simulink model "PrkgClimaPreProcMgr"  (R2022b)
settings: Solver=FixedStepDiscrete  StartTime=0.0  StopTime=10.0  FixedStep=0.2  RelTol=1e-3
(part 1 of 2)
settings Model: Creator=blue.ge  LastModifiedBy=blue.ge
settings DataIOCC: SaveFormat=StructureWithTime
settings OptimizationCC: DefaultParameterBehavior=Inlined  EnhancedBackFolding=on  PassReuseOutputArgsAs=Individual arguments  ZeroExternalMemoryAtStartup=off  ZeroInternalMemoryAtStartup=off  OptimizeBlockOrder=speed  DifferentSizesBufferReuse=on  OptimizationCustomize=off  EfficientTunableParamExpr=on
settings DebuggingCC: ParameterTunabilityLossMsg=error
settings ModelReferenceCC: ModelReferenceNumInstancesAllowed=Single
settings SFSimCC: MATLABDynamicMemAlloc=off
settings RTWCC: Description=AUTOSAR  SystemTargetFile=autosar.tlc  GenCodeOnly=on  TemplateMakefile=ert_default_tmf
settings CodeAppCC: ForceParamTrailComments=on  IgnoreCustomStorageClasses=off  OperatorAnnotations=on  SimulinkDataObjDesc=on  SFDataObjDesc=on  InsertBlockDesc=on
settings STFCustomTargetCC: TargetLangStandard=C99 (ISO)  MultiwordLength=256  ParMdlRefBuildCompliant=on  CompOptLevelCompliant=on  IncludeMdlTerminateFcn=off  CombineOutputUpdateFcns=on  SuppressErrorStatus=on  ERTCustomFileBanners=on  SupportNonFinite=off  SupportComplex=off  SupportContinuousTime=off  SupportNonInlinedSFcns=off  RemoveResetFunc=on  MATLABClassNameForMDSCustomization=Simulink.SoftwareTarget.ERTCustomization  AutosarCompliant=on  UseToolchainInfoCompliant=on  LookupTableObjectStructAxisOrder=2,1,3,4,...  LUTObjectStructOrderEvenSpacing=Size,Table,Breakpoints  SystemTargetFile=autosar.tlc
settings ERTTargetCC: TargetLangStandard=C99 (ISO)  ConcurrentExecutionCompliant=off  IncludeMdlTerminateFcn=off  SuppressErrorStatus=on  SupportNonFinite=off  SupportComplex=off  ModelStepFunctionPrototypeControlCompliant=off  CPPClassGenCompliant=off  AutosarCompliant=on  LookupTableObjectStructAxisOrder=2,1,3,4,...  LUTObjectStructOrderEvenSpacing=Size,Table,Breakpoints  InlinedParameterPlacement=NonHierarchical  ERTCustomFileTemplate=example_file_process.tlc

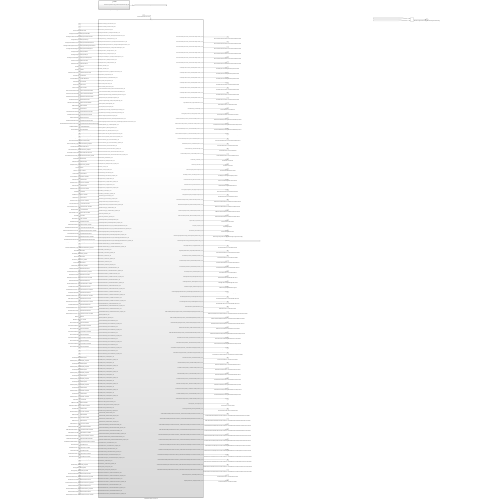
[thumbnail: Simulink's stored preview of the top-level diagram]
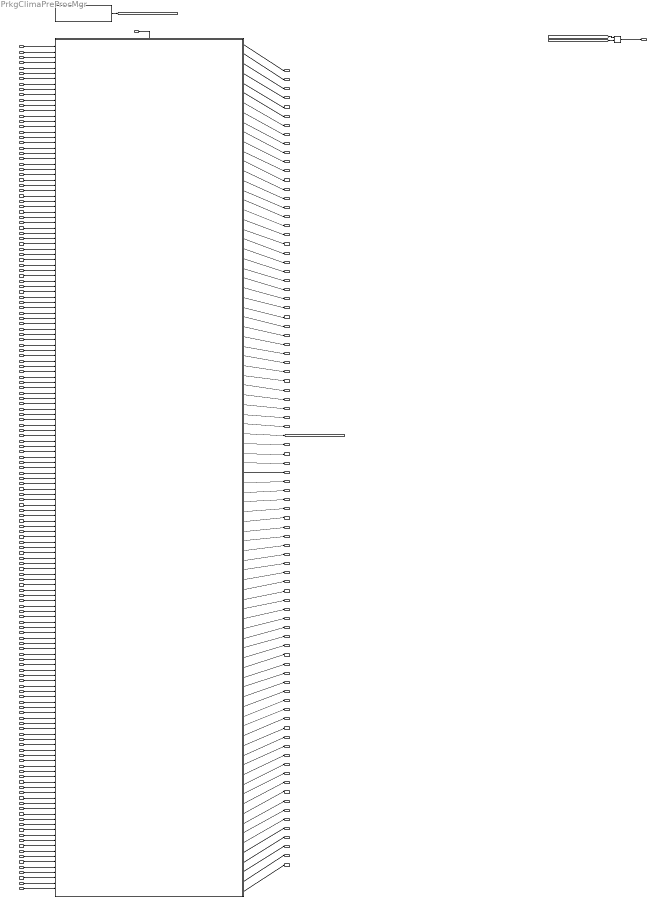
== system PrkgClimaPreProcMgr ==
  PrkgClimaPreProcMgr_Runnable : Inport  SampleTime=0.2  OutputFunctionCall=on
  AmbTFildForClimaNgcc_AmbTFildForClima : Inport  Port=2  OutDataTypeStr=T30  PortDimensions=1
  AmbTFildForClimaQf_AmbTFildForClimaQf : Inport  Port=3  OutDataTypeStr=Enum: GenQf1  PortDimensions=1
  CarTiIntForClima_CarTiIntForClima : Inport  Port=4  OutDataTypeStr=CarTiGlb  PortDimensions=1
  ClimaCfgForClimaCtrlMan1_ClimaCfgForClimaCtrlMan1 : Inport  Port=5  OutDataTypeStr=Boolean  PortDimensions=1
  ClimaCfgForComIfGenn2ForTelm1_ClimaCfgForComIfGenn2ForTelm1 : Inport  Port=6  OutDataTypeStr=Boolean  PortDimensions=1
  ClimaCfgForHeatrTyp1_ClimaCfgForHeatrTyp1 : Inport  Port=7  OutDataTypeStr=Enum: ClimaCfgForHeatrTyp1  PortDimensions=1
  ClimaCfgForHvacHeatrElecFrntPrsnt1_ClimaCfgForHvacHeatrElecFrntPrsnt1 : Inport  Port=8  OutDataTypeStr=Boolean  PortDimensions=1
  ClimaCfgForSeatHeatgForRowFirstPrsnt1_ClimaCfgForSeatHeatgForRowFirstPrsnt1 : Inport  Port=9  OutDataTypeStr=Boolean  PortDimensions=1
  ClimaCfgForSteerWhlHeatgPrsnt1_ClimaCfgForSteerWhlHeatgPrsnt1 : Inport  Port=10  OutDataTypeStr=Boolean  PortDimensions=1
  ClimaCfgForVehElec1_ClimaCfgForVehElec1 : Inport  Port=11  OutDataTypeStr=Boolean  PortDimensions=1
  ClimaCfgForVehHyb1_ClimaCfgForVehHyb1 : Inport  Port=12  OutDataTypeStr=Boolean  PortDimensions=1
  ClimaCfgForVrntOfVehSysElec1_ClimaCfgForVrntOfVehSysElec1 : Inport  Port=13  OutDataTypeStr=Enum: ClimaCenCfgForVrntOfVehSysElec1  PortDimensions=1
  ClimaRqrd1ConnGen2_ClimaRqrd1ConnGen2 : Inport  Port=14  OutDataTypeStr=Enum: OnOffNoReq  PortDimensions=1
  ClimaRqrdFromRem1_ClimaRqrdFromRem1 : Inport  Port=15  OutDataTypeStr=Enum: OnOffNoReq  PortDimensions=1
  ClimaStrtTi_ClimaStrtTi : Inport  Port=16  OutDataTypeStr=CarTiGlb  PortDimensions=1
  ClimaWarn_ClimaWarn : Inport  Port=17  OutDataTypeStr=Enum: ClimaWarn  PortDimensions=1
  ClngRqrdFromTelmConnGen2_ClngRqrdFromTelmConnGen2 : Inport  Port=18  OutDataTypeStr=Enum: OnOffNoReq  PortDimensions=1
  CmptmtObsvrSts_CmptmtObsvrSts : Inport  Port=19  OutDataTypeStr=Enum: ObsvrStsForCmptmt  PortDimensions=1
  CmptmtSunMtrlTEstimd_CmptmtSunMtrlTEstimd : Inport  Port=20  OutDataTypeStr=T30  PortDimensions=1
  DCChrgnHndlSts_DCChrgnHndlSts : Inport  Port=21  OutDataTypeStr=Enum: DCChrgnHndlStsE2E  PortDimensions=1
  DshbTForClima_DshbTForClima : Inport  Port=22  OutDataTypeStr=T30  PortDimensions=1
  DshbTForClimaQf_DshbTForClimaQf : Inport  Port=23  OutDataTypeStr=Enum: DshbTForClimaQf  PortDimensions=1
  HvacAirTForRowFirstAtVentLeRaw_HvacAirTForRowFirstAtVentLeRaw : Inport  Port=24  OutDataTypeStr=Bus: HvacAirTForRowFirstAtVentLe  PortDimensions=1  BusOutputAsStruct=on
  HvacAirTForRowFirstAtVentRiRaw_HvacAirTForRowFirstAtVentRiRaw : Inport  Port=25  OutDataTypeStr=Bus: HvacAirTForRowFirstAtVentRi  PortDimensions=1  BusOutputAsStruct=on
  MemReadForPrkgClimaPreProcMgr_MemReadForPrkgClimaPreProcMgr : Inport  Port=26  OutDataTypeStr=Bus: MemRecForPrkgClimaPreProcMgr  PortDimensions=1  BusOutputAsStruct=on
  MemReqStsForPrkgClima_MemReqStsForPrkgClima : Inport  Port=27  OutDataTypeStr=Enum: MemReqSts  PortDimensions=1
  NetReqFromPrkgClimaEveMgr_NetReqFromPrkgClimaEveMgr : Inport  Port=28  OutDataTypeStr=Boolean  PortDimensions=1
  OnBdChrgrHndlSts_OnBdChrgrHndlSts : Inport  Port=29  OutDataTypeStr=Enum: OnBdChrgrSts1  PortDimensions=1
  ReqForPrkgClima_ReqForPrkgClima : Inport  Port=30  OutDataTypeStr=Enum: PrkgClimaReqSts  PortDimensions=1
  ReqForSwtHeatrTypFromActvMgr_ReqForSwtHeatrTypFromActvMgr : Inport  Port=31  OutDataTypeStr=Boolean  PortDimensions=1
  ReqForSwtHeatrTypFromEveMgr_ReqForSwtHeatrTypFromEveMgr : Inport  Port=32  OutDataTypeStr=Boolean  PortDimensions=1
  RtMaxForPrePrkgClima_RtMaxForPrePrkgClima : Inport  Port=33  OutDataTypeStr=Ti33  PortDimensions=1
  SeatHeatDurgClimaEnadConnGen2_SeatHeatDurgClimaEnadConnGen2 : Inport  Port=34  OutDataTypeStr=Enum: SeatHeatDurgClimaEnad  PortDimensions=1
  SteerWhlHeatgDurgClimEandFromTelmConnGen2_SteerWhlHeatgDurgClimEandFromTelmConnGen2 : Inport  Port=35  OutDataTypeStr=Enum: OnOff1  PortDimensions=1
  VehModMngtGlbSafe1_Ngcc_VehModMngtGlbSafe1 : Inport  Port=36  OutDataTypeStr=Bus: VehModMngtGlbSafe1  PortDimensions=1  BusOutputAsStruct=on
  WarnForEgyAllwdLimd_WarnForEgyAllwdLimd : Inport  Port=37  OutDataTypeStr=Boolean  PortDimensions=1
  WarnForHybWoUForTmr_WarnForHybWoUForTmr : Inport  Port=38  OutDataTypeStr=Boolean  PortDimensions=1
  WarnForHybWoUFromActvMgr_WarnForHybWoUFromActvMgr : Inport  Port=39  OutDataTypeStr=Boolean  PortDimensions=1
  WarnForTmrPreCdngFctNotAvl_WarnForTmrPreCdngFctNotAvl : Inport  Port=40  OutDataTypeStr=Boolean  PortDimensions=1
  BookTrvlClimaActvReq_Ngcc_BookTrvlClimaActvReq : Inport  Port=41  OutDataTypeStr=Enum: OnOffNoReq  PortDimensions=1
  BookTrvlClimaActvReq_Ngcc_BookTrvlClimaActvReq_IsUpdated : Inport  Port=42  OutDataTypeStr=boolean  PortDimensions=1
  CbnOverHeatProtnEna_CbnOverHeatProtnEna : Inport  Port=43  OutDataTypeStr=Enum: OnOff1  PortDimensions=1
  CbnOverHeatProtnEna_CbnOverHeatProtnEna_IsUpdated : Inport  Port=44  OutDataTypeStr=boolean  PortDimensions=1
  CbnOverHeatProtnEnaFromTelm_CbnOverHeatProtnEnaFromTelm : Inport  Port=45  OutDataTypeStr=Enum: OnOff1  PortDimensions=1
  CbnOverHeatProtnEnaFromTelm_CbnOverHeatProtnEnaFromTelm_IsUpdated : Inport  Port=46  OutDataTypeStr=boolean  PortDimensions=1
  CdsClimaOnReq_CdsClimaOnReq : Inport  Port=47  OutDataTypeStr=Enum: OnOff1  PortDimensions=1
  ClimaRqrdFromHmi_ClimaRqrdFromHmi : Inport  Port=48  OutDataTypeStr=Enum: OnOffNoReq  PortDimensions=1
  ClimaRqrdFromHmi_ClimaRqrdFromHmi_IsUpdated : Inport  Port=49  OutDataTypeStr=boolean  PortDimensions=1
  ClimaTmr_ClimaTmr : Inport  Port=50  OutDataTypeStr=CarTiGlb  PortDimensions=1
  ClimaTmr_ClimaTmr_IsUpdated : Inport  Port=51  OutDataTypeStr=boolean  PortDimensions=1
  ClimaTmrStsRqrd_ClimaTmrStsRqrd : Inport  Port=52  OutDataTypeStr=Enum: OnOff1  PortDimensions=1
  ClimaTmrStsRqrd_ClimaTmrStsRqrd_IsUpdated : Inport  Port=53  OutDataTypeStr=boolean  PortDimensions=1
  ClngRqrdFromHmi_ClngRqrdFromHmi : Inport  Port=54  OutDataTypeStr=Enum: OnOffNoReq  PortDimensions=1
  ClngRqrdFromHmi_ClngRqrdFromHmi_IsUpdated : Inport  Port=55  OutDataTypeStr=boolean  PortDimensions=1
  ClngRqrdFromTelm_ClngRqrdFromTelm : Inport  Port=56  OutDataTypeStr=Enum: OnOffNoReq  PortDimensions=1
  ClngRqrdFromTelm_ClngRqrdFromTelm_IsUpdated : Inport  Port=57  OutDataTypeStr=boolean  PortDimensions=1
  ErsCmdByKey_ErsCmdByKey : Inport  Port=58  OutDataTypeStr=Enum: ErsCmd  PortDimensions=1
  ErsCmdByKey_ErsCmdByKey_IsUpdated : Inport  Port=59  OutDataTypeStr=boolean  PortDimensions=1
  HeatrPreCdngTyp_HeatrPreCdngTyp : Inport  Port=60  OutDataTypeStr=Enum: HeatrPreCdngTyp  PortDimensions=1
  HeatrPreCdngTyp_HeatrPreCdngTyp_IsUpdated : Inport  Port=61  OutDataTypeStr=boolean  PortDimensions=1
  IncomingCO2CtrlFromHmi_IncomingCO2CtrlFromHmi : Inport  Port=62  OutDataTypeStr=Enum: OnOff1  PortDimensions=1
  IncomingCO2CtrlFromHmi_IncomingCO2CtrlFromHmi_IsUpdated : Inport  Port=63  OutDataTypeStr=boolean  PortDimensions=1
  RemStrtHvCtrlReq_Ngcc_RemStrtHvCtrlReq : Inport  Port=64  OutDataTypeStr=Bus: RemStrHvCtrlReq  PortDimensions=1  BusOutputAsStruct=on
  RemStrtHvCtrlReq_Ngcc_RemStrtHvCtrlReq_IsUpdated : Inport  Port=65  OutDataTypeStr=boolean  PortDimensions=1
  RemVentReq_RemVentReq : Inport  Port=66  OutDataTypeStr=Enum: OnOffNoReq  PortDimensions=1
  RemVentReq_RemVentReq_IsUpdated : Inport  Port=67  OutDataTypeStr=boolean  PortDimensions=1
  SeatHeatDurgClimaEnad_SeatHeatDurgClimaEnad : Inport  Port=68  OutDataTypeStr=Enum: SeatHeatDurgClimaEnad  PortDimensions=1
  SeatHeatDurgClimaEnad_SeatHeatDurgClimaEnad_IsUpdated : Inport  Port=69  OutDataTypeStr=boolean  PortDimensions=1
  SeatHeatDurgClimaEnadFromTelm_Ngcc_SeatHeatDurgClimaEnadFromTelm : Inport  Port=70  OutDataTypeStr=Enum: SeatHeatDurgClimaEnad  PortDimensions=1
  SeatHeatDurgClimaEnadFromTelm_Ngcc_SeatHeatDurgClimaEnadFromTelm_IsUpdated : Inport  Port=71  OutDataTypeStr=boolean  PortDimensions=1
  SteerWhlHeatgDurgClimaEnad_SteerWhlHeatgDurgClimaEnad : Inport  Port=72  OutDataTypeStr=Enum: OnOff1  PortDimensions=1
  SteerWhlHeatgDurgClimaEnad_SteerWhlHeatgDurgClimaEnad_IsUpdated : Inport  Port=73  OutDataTypeStr=boolean  PortDimensions=1
  SteerWhlHeatgDurgClimaEnadFromTelm_SteerWhlHeatgDurgClimaEnadFromTelm : Inport  Port=74  OutDataTypeStr=Enum: OnOff1  PortDimensions=1
  SteerWhlHeatgDurgClimaEnadFromTelm_SteerWhlHeatgDurgClimaEnadFromTelm_IsUpdated : Inport  Port=75  OutDataTypeStr=boolean  PortDimensions=1
  StrtRemSts1WdStrtRemSts_Ngcc_StrtRemSts1WdStrtRemSts : Inport  Port=76  OutDataTypeStr=Enum: StrtRemSts1  PortDimensions=1
  StrtRemSts1WdStrtRemSts_Ngcc_StrtRemSts1WdStrtRemSts_IsUpdated : Inport  Port=77  OutDataTypeStr=boolean  PortDimensions=1
  TelmClimaReq_TelmClimaReq : Inport  Port=78  OutDataTypeStr=Enum: OnOffNoReq  PortDimensions=1
  TelmClimaReq_TelmClimaReq_IsUpdated : Inport  Port=79  OutDataTypeStr=boolean  PortDimensions=1
  TelmClimaTmr_TelmClimaTmr : Inport  Port=80  OutDataTypeStr=TimeDesForSWDL  PortDimensions=1
  TelmClimaTmr_TelmClimaTmr_IsUpdated : Inport  Port=81  OutDataTypeStr=boolean  PortDimensions=1
  TelmClimaTSet_TelmClimaTSet : Inport  Port=82  OutDataTypeStr=Bus: TelmClimaTSet  PortDimensions=1  BusOutputAsStruct=on
  TelmClimaTSet_TelmClimaTSet_IsUpdated : Inport  Port=83  OutDataTypeStr=boolean  PortDimensions=1
  TelmSeatDrvHeatClimaLvl_TelmSeatDrvHeatClimaLvl : Inport  Port=84  OutDataTypeStr=Enum: TelmSeatClimaLvl  PortDimensions=1
  TelmSeatDrvHeatClimaLvl_TelmSeatDrvHeatClimaLvl_IsUpdated : Inport  Port=85  OutDataTypeStr=boolean  PortDimensions=1
  TelmSeatDrvVentnClimaLvl_TelmSeatDrvVentnClimaLvl : Inport  Port=86  OutDataTypeStr=Enum: TelmSeatClimaLvl  PortDimensions=1
  TelmSeatDrvVentnClimaLvl_TelmSeatDrvVentnClimaLvl_IsUpdated : Inport  Port=87  OutDataTypeStr=boolean  PortDimensions=1
  TelmSeatPassHeatClimaLvl_TelmSeatPassHeatClimaLvl : Inport  Port=88  OutDataTypeStr=Enum: TelmSeatClimaLvl  PortDimensions=1
  TelmSeatPassHeatClimaLvl_TelmSeatPassHeatClimaLvl_IsUpdated : Inport  Port=89  OutDataTypeStr=boolean  PortDimensions=1
  TelmSeatPassVentnClimaLvl_TelmSeatPassVentnClimaLvl : Inport  Port=90  OutDataTypeStr=Enum: TelmSeatClimaLvl  PortDimensions=1
  TelmSeatPassVentnClimaLvl_TelmSeatPassVentnClimaLvl_IsUpdated : Inport  Port=91  OutDataTypeStr=boolean  PortDimensions=1
  TelmSeatSecLeHeatClimaLvl_TelmSeatSecLeHeatClimaLvl : Inport  Port=92  OutDataTypeStr=Enum: TelmSeatClimaLvl  PortDimensions=1
  TelmSeatSecLeHeatClimaLvl_TelmSeatSecLeHeatClimaLvl_IsUpdated : Inport  Port=93  OutDataTypeStr=boolean  PortDimensions=1
  TelmSeatSecLeVentnClimaLvl_TelmSeatSecLeVentnClimaLvl : Inport  Port=94  OutDataTypeStr=Enum: TelmSeatClimaLvl  PortDimensions=1
  TelmSeatSecLeVentnClimaLvl_TelmSeatSecLeVentnClimaLvl_IsUpdated : Inport  Port=95  OutDataTypeStr=boolean  PortDimensions=1
  TelmSeatSecRiHeatClimaLvl_TelmSeatSecRiHeatClimaLvl : Inport  Port=96  OutDataTypeStr=Enum: TelmSeatClimaLvl  PortDimensions=1
  TelmSeatSecRiHeatClimaLvl_TelmSeatSecRiHeatClimaLvl_IsUpdated : Inport  Port=97  OutDataTypeStr=boolean  PortDimensions=1
  TelmSeatSecRiVentnClimaLvl_TelmSeatSecRiVentnClimaLvl : Inport  Port=98  OutDataTypeStr=Enum: TelmSeatClimaLvl  PortDimensions=1
  TelmSeatSecRiVentnClimaLvl_TelmSeatSecRiVentnClimaLvl_IsUpdated : Inport  Port=99  OutDataTypeStr=boolean  PortDimensions=1
  HMIExtVent_HMIExtVent : Inport  Port=100  OutDataTypeStr=Bus: HmiExternaVent  PortDimensions=1  BusOutputAsStruct=on
  HMIExtVent_HMIExtVent_IsUpdated : Inport  Port=101  OutDataTypeStr=boolean  PortDimensions=1
  BookTrvlClimaActvReq2_BookTrvlClimaActvReq2 : Inport  Port=102  OutDataTypeStr=Enum: OnOffNoReq  PortDimensions=1
  BookTrvlClimaActvReq2_BookTrvlClimaActvReq2_IsUpdated : Inport  Port=103  OutDataTypeStr=boolean  PortDimensions=1
  BookTrvlClimaActvReq3_BookTrvlClimaActvReq3 : Inport  Port=104  OutDataTypeStr=Enum: OnOffNoReq  PortDimensions=1
  BookTrvlClimaActvReq3_BookTrvlClimaActvReq3_IsUpdated : Inport  Port=105  OutDataTypeStr=boolean  PortDimensions=1
  BookTrvlClimaActvReq4_BookTrvlClimaActvReq4 : Inport  Port=106  OutDataTypeStr=Enum: OnOffNoReq  PortDimensions=1
  BookTrvlClimaActvReq4_BookTrvlClimaActvReq4_IsUpdated : Inport  Port=107  OutDataTypeStr=boolean  PortDimensions=1
  BookTrvlClimaActvReq5_BookTrvlClimaActvReq5 : Inport  Port=108  OutDataTypeStr=Enum: OnOffNoReq  PortDimensions=1
  BookTrvlClimaActvReq5_BookTrvlClimaActvReq5_IsUpdated : Inport  Port=109  OutDataTypeStr=boolean  PortDimensions=1
  BookTrvlClimaActvReq6_BookTrvlClimaActvReq6 : Inport  Port=110  OutDataTypeStr=Enum: OnOffNoReq  PortDimensions=1
  BookTrvlClimaActvReq6_BookTrvlClimaActvReq6_IsUpdated : Inport  Port=111  OutDataTypeStr=boolean  PortDimensions=1
  BookTrvlClimaActvReq7_BookTrvlClimaActvReq7 : Inport  Port=112  OutDataTypeStr=Enum: OnOffNoReq  PortDimensions=1
  BookTrvlClimaActvReq7_BookTrvlClimaActvReq7_IsUpdated : Inport  Port=113  OutDataTypeStr=boolean  PortDimensions=1
  ClimaWakUpTiDif1_ClimaWakUpTiDif1 : Inport  Port=114  OutDataTypeStr=Mins2  PortDimensions=1
  ClimaWakUpTiDif1_ClimaWakUpTiDif1_IsUpdated : Inport  Port=115  OutDataTypeStr=boolean  PortDimensions=1
  ClimaWakUpTiDif2_ClimaWakUpTiDif2 : Inport  Port=116  OutDataTypeStr=Mins2  PortDimensions=1
  ClimaWakUpTiDif2_ClimaWakUpTiDif2_IsUpdated : Inport  Port=117  OutDataTypeStr=boolean  PortDimensions=1
  ClimaWakUpTiDif3_ClimaWakUpTiDif3 : Inport  Port=118  OutDataTypeStr=Mins2  PortDimensions=1
  ClimaWakUpTiDif3_ClimaWakUpTiDif3_IsUpdated : Inport  Port=119  OutDataTypeStr=boolean  PortDimensions=1
  ClimaWakUpTiDif4_ClimaWakUpTiDif4 : Inport  Port=120  OutDataTypeStr=Mins2  PortDimensions=1
  ClimaWakUpTiDif4_ClimaWakUpTiDif4_IsUpdated : Inport  Port=121  OutDataTypeStr=boolean  PortDimensions=1
  ClimaWakUpTiDif5_ClimaWakUpTiDif5 : Inport  Port=122  OutDataTypeStr=Mins2  PortDimensions=1
  ClimaWakUpTiDif5_ClimaWakUpTiDif5_IsUpdated : Inport  Port=123  OutDataTypeStr=boolean  PortDimensions=1
  ClimaWakUpTiDif6_ClimaWakUpTiDif6 : Inport  Port=124  OutDataTypeStr=Mins2  PortDimensions=1
  ClimaWakUpTiDif6_ClimaWakUpTiDif6_IsUpdated : Inport  Port=125  OutDataTypeStr=boolean  PortDimensions=1
  ClimaWakUpTiDif7_ClimaWakUpTiDif7 : Inport  Port=126  OutDataTypeStr=Mins2  PortDimensions=1
  ClimaWakUpTiDif7_ClimaWakUpTiDif7_IsUpdated : Inport  Port=127  OutDataTypeStr=boolean  PortDimensions=1
  EngSt1WdSts_Ngcc_EngSt1WdSts : Inport  Port=128  OutDataTypeStr=Enum: EngSt1  PortDimensions=1
  HMIExtVentonoffReq_HMIExtVentonoffReq : Inport  Port=129  OutDataTypeStr=Enum: OnOffNoReq  PortDimensions=1
  HMIExtVentonoffReq_HMIExtVentonoffReq_IsUpdated : Inport  Port=130  OutDataTypeStr=boolean  PortDimensions=1
  RemStrtExtnTiReq_RemStrtExtnTiReq : Inport  Port=131  OutMin=0  OutMax=59  OutDataTypeStr=Mins1  PortDimensions=1
  RemStrtExtnTiReq_RemStrtExtnTiReq_IsUpdated : Inport  Port=132  OutDataTypeStr=boolean  PortDimensions=1
  TelmBookClimaReq_TelmBookClimaReq : Inport  Port=133  OutDataTypeStr=Bus: TelmBookClimaReq  PortDimensions=1  BusOutputAsStruct=on
  TelmBookClimaReq_TelmBookClimaReq_IsUpdated : Inport  Port=134  OutDataTypeStr=boolean  PortDimensions=1
  TelmBookClimaTmr_TelmBookClimaTmr : Inport  Port=135  OutDataTypeStr=Ti33  PortDimensions=1
  TelmBookClimaTmr_TelmBookClimaTmr_IsUpdated : Inport  Port=136  OutDataTypeStr=boolean  PortDimensions=1
  TelmBookSeatHeatClimalReq_TelmBookSeatHeatClimalReq : Inport  Port=137  OutDataTypeStr=Bus: TelmBookSeatHeatClimalReq  PortDimensions=1  BusOutputAsStruct=on
  TelmBookSeatHeatClimalReq_TelmBookSeatHeatClimalReq_IsUpdated : Inport  Port=138  OutDataTypeStr=boolean  PortDimensions=1
  TelmBookSeatVentnClimalReq_TelmBookSeatVentnClimalReq : Inport  Port=139  OutDataTypeStr=Bus: TelmBookSeatVentnClimalReq  PortDimensions=1  BusOutputAsStruct=on
  TelmBookSeatVentnClimalReq_TelmBookSeatVentnClimalReq_IsUpdated : Inport  Port=140  OutDataTypeStr=boolean  PortDimensions=1
  TelmBookSteerWhlHeatClimalReq_TelmBookSteerWhlHeatClimalReq : Inport  Port=141  OutDataTypeStr=Enum: OnOff1  PortDimensions=1
  TelmBookSteerWhlHeatClimalReq_TelmBookSteerWhlHeatClimalReq_IsUpdated : Inport  Port=142  OutDataTypeStr=boolean  PortDimensions=1
  TelmClimaExtVentnLvl_TelmClimaExtVentnLvl : Inport  Port=143  OutDataTypeStr=Enum: HmiHvacFanLvl  PortDimensions=1
  TelmClimaExtVentnLvl_TelmClimaExtVentnLvl_IsUpdated : Inport  Port=144  OutDataTypeStr=boolean  PortDimensions=1
  TelmClimaExtVentnReq_TelmClimaExtVentnReq : Inport  Port=145  OutDataTypeStr=Enum: OnOffNoReq  PortDimensions=1
  TelmClimaExtVentnReq_TelmClimaExtVentnReq_IsUpdated : Inport  Port=146  OutDataTypeStr=boolean  PortDimensions=1
  TelmClimaExtVentnTempSet_TelmClimaExtVentnTempSet : Inport  Port=147  OutDataTypeStr=Bus: TelmClimaTSet  PortDimensions=1  BusOutputAsStruct=on
  TelmClimaExtVentnTempSet_TelmClimaExtVentnTempSet_IsUpdated : Inport  Port=148  OutDataTypeStr=boolean  PortDimensions=1
  TelmDefrostReq_TelmDefrostReq : Inport  Port=149  OutDataTypeStr=Enum: OnOffNoReq  PortDimensions=1
  TelmDefrostReq_TelmDefrostReq_IsUpdated : Inport  Port=150  OutDataTypeStr=boolean  PortDimensions=1
  TelmOxygenReq_TelmOxygenReq : Inport  Port=151  OutDataTypeStr=Enum: OnOffNoReq  PortDimensions=1
  TelmOxygenReq_TelmOxygenReq_IsUpdated : Inport  Port=152  OutDataTypeStr=boolean  PortDimensions=1
  TelmSeatThrdLeHeatClimaLvl_TelmSeatThrdLeHeatClimaLvl : Inport  Port=153  OutDataTypeStr=Enum: TelmSeatClimaLvl  PortDimensions=1
  TelmSeatThrdLeHeatClimaLvl_TelmSeatThrdLeHeatClimaLvl_IsUpdated : Inport  Port=154  OutDataTypeStr=boolean  PortDimensions=1
  TelmSeatThrdLeVentnClimaLvl_TelmSeatThrdLeVentnClimaLvl : Inport  Port=155  OutDataTypeStr=Enum: TelmSeatClimaLvl  PortDimensions=1
  TelmSeatThrdLeVentnClimaLvl_TelmSeatThrdLeVentnClimaLvl_IsUpdated : Inport  Port=156  OutDataTypeStr=boolean  PortDimensions=1
  TelmSeatThrdRiHeatClimaLvl_TelmSeatThrdRiHeatClimaLvl : Inport  Port=157  OutDataTypeStr=Enum: TelmSeatClimaLvl  PortDimensions=1
  TelmSeatThrdRiHeatClimaLvl_TelmSeatThrdRiHeatClimaLvl_IsUpdated : Inport  Port=158  OutDataTypeStr=boolean  PortDimensions=1
  TelmSeatThrdRiVentnClimaLvl_TelmSeatThrdRiVentnClimaLvl : Inport  Port=159  OutDataTypeStr=Enum: TelmSeatClimaLvl  PortDimensions=1
  TelmSeatThrdRiVentnClimaLvl_TelmSeatThrdRiVentnClimaLvl_IsUpdated : Inport  Port=160  OutDataTypeStr=boolean  PortDimensions=1
  MemWrForPrkgClimaPreProcMgr_MemWrForPrkgClimaPreProcMgr_merge : Merge
  PrkgClimaPreProcMgr_Init : Subsystem
  PrkgClimaPreProcMgr_Runnable_sys : Subsystem
  MemWrForPrkgClimaPreProcMgr_MemWrForPrkgClimaPreProcMgr : Outport  OutDataTypeStr=Bus: MemRecForPrkgClimaPreProcMgr  PortDimensions=1  BusOutputAsStruct=on
  BookTrvlClimaActvReq2FromExSrc_BookTrvlClimaActvReq2FromExSrc : Outport  Port=2  OutDataTypeStr=Enum: OnOffNoReq  PortDimensions=1
  BookTrvlClimaActvReq3FromExSrc_BookTrvlClimaActvReq3FromExSrc : Outport  Port=3  OutDataTypeStr=Enum: OnOffNoReq  PortDimensions=1
  BookTrvlClimaActvReq4FromExSrc_BookTrvlClimaActvReq4FromExSrc : Outport  Port=4  OutDataTypeStr=Enum: OnOffNoReq  PortDimensions=1
  BookTrvlClimaActvReq5FromExSrc_BookTrvlClimaActvReq5FromExSrc : Outport  Port=5  OutDataTypeStr=Enum: OnOffNoReq  PortDimensions=1
  BookTrvlClimaActvReq6FromExSrc_BookTrvlClimaActvReq6FromExSrc : Outport  Port=6  OutDataTypeStr=Enum: OnOffNoReq  PortDimensions=1
  BookTrvlClimaActvReq7FromExSrc_BookTrvlClimaActvReq7FromExSrc : Outport  Port=7  OutDataTypeStr=Enum: OnOffNoReq  PortDimensions=1
  ClimaWakUpTiDif1FromExSrc_ClimaWakUpTiDif1FromExSrc : Outport  Port=8  OutDataTypeStr=Mins2  PortDimensions=1
  ClimaWakUpTiDif2FromExSrc_ClimaWakUpTiDif2FromExSrc : Outport  Port=9  OutDataTypeStr=Mins2  PortDimensions=1
  ClimaWakUpTiDif3FromExSrc_ClimaWakUpTiDif3FromExSrc : Outport  Port=10  OutDataTypeStr=Mins2  PortDimensions=1
  ClimaWakUpTiDif4FromExSrc_ClimaWakUpTiDif4FromExSrc : Outport  Port=11  OutDataTypeStr=Mins2  PortDimensions=1
  ClimaWakUpTiDif5FromExSrc_ClimaWakUpTiDif5FromExSrc : Outport  Port=12  OutDataTypeStr=Mins2  PortDimensions=1
  ClimaWakUpTiDif6FromExSrc_ClimaWakUpTiDif6FromExSrc : Outport  Port=13  OutDataTypeStr=Mins2  PortDimensions=1
  ClimaWakUpTiDif7FromExSrc_ClimaWakUpTiDif7FromExSrc : Outport  Port=14  OutDataTypeStr=Mins2  PortDimensions=1
  EngSt1WdStsFromExsrc_EngSt1WdStsFromExsrc : Outport  Port=15  OutDataTypeStr=Enum: EngSt1  PortDimensions=1
  RemClimaHvRspn_RemClimaHvRspn : Outport  Port=16  OutDataTypeStr=Enum: OnOff1  PortDimensions=1
  TelmOxygenReqFromExSrc_TelmOxygenReqFromExSrc : Outport  Port=17  OutDataTypeStr=Enum: OnOffNoReq  PortDimensions=1
  TelmSeatThrdLeHeatReqFromExsrc_TelmSeatThrdLeHeatReqFromExsrc : Outport  Port=18  OutDataTypeStr=Enum: TelmSeatClimaLvlExsrc  PortDimensions=1
  TelmSeatThrdLeVentnReqFromExsrc_TelmSeatThrdLeVentnReqFromExsrc : Outport  Port=19  OutDataTypeStr=Enum: TelmSeatClimaLvlExsrc  PortDimensions=1
  TelmSeatThrdRiHeatReqFromExsrc_TelmSeatThrdRiHeatReqFromExsrc : Outport  Port=20  OutDataTypeStr=Enum: TelmSeatClimaLvlExsrc  PortDimensions=1
  TelmSeatThrdRiVentnReqFromExsrc_TelmSeatThrdRiVentnReqFromExsrc : Outport  Port=21  OutDataTypeStr=Enum: TelmSeatClimaLvlExsrc  PortDimensions=1
  BookTrvlClimaActvReqfromExsrc_BookTrvlClimaActvReqfromExsrc : Outport  Port=22  OutDataTypeStr=Enum: OnOffNoReq  PortDimensions=1
  CdsClimaOnReqfromExsrc_CdsClimaOnReqfromExsrc : Outport  Port=23  OutDataTypeStr=Enum: OnOff1  PortDimensions=1
  ClimaOvrHeatProRspn_ClimaOvrHeatProRspn : Outport  Port=24  OutDataTypeStr=Enum: OnOff1  PortDimensions=1
  ClimaOvrHeatReqFromExrsc_ClimaOvrHeatReqFromExrsc : Outport  Port=25  OutDataTypeStr=Enum: PreCdngReqFromExtSrc  PortDimensions=1
  ClimaStrtTiDly_ClimaStrtTiDly : Outport  Port=26  OutDataTypeStr=Ti39  PortDimensions=1
  ClimaTmrSts_ClimaTmrSts : Outport  Port=27  OutDataTypeStr=Enum: OnOff1  PortDimensions=1
  DshbTForPrkgClima_DshbTForPrkgClima : Outport  Port=28  OutDataTypeStr=T30  PortDimensions=1
  ErsCmdByKeyFromExsrc_ErsCmdByKeyFromExsrc : Outport  Port=29  OutDataTypeStr=Enum: ErsCmd  PortDimensions=1
  ErsRunTimeFromExtSrc_ErsRunTimeFromExtSrc : Outport  Port=30  OutDataTypeStr=Ti39  PortDimensions=1
  HeatrElecNotAvlForTmr_HeatrElecNotAvlForTmr : Outport  Port=31  OutDataTypeStr=Boolean  PortDimensions=1
  HeatrTypSetgForPrkgClima_HeatrTypSetgForPrkgClima : Outport  Port=32  OutDataTypeStr=Enum: HeatrPreCdngTyp  PortDimensions=1
  HeatUpRqrdForPrkgClima_HeatUpRqrdForPrkgClima : Outport  Port=33  OutDataTypeStr=Boolean  PortDimensions=1
  HMIExtVentFanVolReqFromExtSrc_HMIExtVentFanVolReqFromExtSrc : Outport  Port=34  OutDataTypeStr=Enum: FanVolReq  PortDimensions=1
  HMIExtVentonoffReqFromExtSrc_HMIExtVentonoffReqFromExtSrc : Outport  Port=35  OutDataTypeStr=Enum: OnOffNoReq  PortDimensions=1
  HMIExtVentTSpReqFromExtSrc_HMIExtVentTSpReqFromExtSrc : Outport  Port=36  OutDataTypeStr=Enum: TSpReq  PortDimensions=1
  HMIExtVentTStsReqFromExtSrc_HMIExtVentTStsReqFromExtSrc : Outport  Port=37  OutMin=-256  OutMax=255.9  OutDataTypeStr=T2  PortDimensions=1
  HvSysCnctWoU_HvSysCnctWoU : Outport  Port=38  OutDataTypeStr=Boolean  PortDimensions=1
  HvSysWoU_HvSysWoU : Outport  Port=39  OutDataTypeStr=Boolean  PortDimensions=1
  IntCo2fromExsrc_IntCo2fromExsrc : Outport  Port=40  OutDataTypeStr=Enum: OnOff1  PortDimensions=1
  MemSaveFlgForPrkgClimaPreProcMgr_MemSaveFlgForPrkgClimaPreProcMgr : Outport  Port=41  OutDataTypeStr=Boolean  PortDimensions=1
  PreClngRqrdFromExtSrc_PreClngRqrdFromExtSrc : Outport  Port=42  OutDataTypeStr=Enum: PreCdngReqFromExtSrc  PortDimensions=1
  PrkgClimaRqrdImdtFromExtSrc_PrkgClimaRqrdImdtFromExtSrc : Outport  Port=43  OutDataTypeStr=Enum: PreCdngReqFromExtSrc  PortDimensions=1
  RemClimaReqFromExtSrc_RemClimaReqFromExtSrc : Outport  Port=44  OutDataTypeStr=Enum: OnOffNoReq  PortDimensions=1
  RemStrtExtnTiReqFromExtSrc_RemStrtExtnTiReqFromExtSrc : Outport  Port=45  OutDataTypeStr=Ti39  PortDimensions=1
  RemStrtHvCtrlReqFromExtSrc_RemStrtHvCtrlReqFromExtSrc : Outport  Port=46  OutDataTypeStr=Enum: ErsCmd  PortDimensions=1
  RemVentReqfromExsrc_RemVentReqfromExsrc : Outport  Port=47  OutDataTypeStr=Enum: OnOffNoReq  PortDimensions=1
  SeatHeatgEnadFromHmi_SeatHeatgEnadFromHmi : Outport  Port=48  OutDataTypeStr=Enum: SeatHeatDurgClimaEnad  PortDimensions=1
  SeatHeatgEnadFromTelm_SeatHeatgEnadFromTelm : Outport  Port=49  OutDataTypeStr=Enum: SeatHeatDurgClimaEnad  PortDimensions=1
  SnsrQfOkForPrkgClima_SnsrQfOkForPrkgClima : Outport  Port=50  OutDataTypeStr=Boolean  PortDimensions=1
  SteerWhlHeatgDurgClimaEnadFromExsrc_SteerWhlHeatgDurgClimaEnadFromExsrc : Outport  Port=51  OutDataTypeStr=Enum: OnOffNoReq  PortDimensions=1
  SteerWhlHeatgEnadFromHmi_SteerWhlHeatgEnadFromHmi : Outport  Port=52  OutDataTypeStr=Boolean  PortDimensions=1
  SteerWhlHeatgEnadFromTelm_SteerWhlHeatgEnadFromTelm : Outport  Port=53  OutDataTypeStr=Boolean  PortDimensions=1
  StrtRemStsFromExsrc_StrtRemStsFromExsrc : Outport  Port=54  OutDataTypeStr=Enum: StrtRemSts1  PortDimensions=1
  TelmBookClimaReqHmiCmptmtTSpSpclFromExtSrc_TelmBookClimaReqHmiCmptmtTSpSpclFromExtSrc : Outport  Port=55  OutDataTypeStr=Enum: NormalLowHigh  PortDimensions=1
  TelmBookClimaReqOnOffNoReqFromExtSrc_TelmBookClimaReqOnOffNoReqFromExtSrc : Outport  Port=56  OutDataTypeStr=Enum: NoReqOnOff  PortDimensions=1
  TelmBookClimaReqTempRangeFromExtSrc_TelmBookClimaReqTempRangeFromExtSrc : Outport  Port=57  OutDataTypeStr=TempRange  PortDimensions=1
  TelmBookClimaTmrFromExtSrc_TelmBookClimaTmrFromExtSrc : Outport  Port=58  OutDataTypeStr=Ti33  PortDimensions=1
  TelmBookSteerWhlHeatClimalReqFromExtSrc_TelmBookSteerWhlHeatClimalReqFromExtSrc : Outport  Port=59  OutDataTypeStr=Enum: OnOffNoReq  PortDimensions=1
  TelmClimaExtVentnLvlFromExtSrc_TelmClimaExtVentnLvlFromExtSrc : Outport  Port=60  OutDataTypeStr=Enum: HmiHvacFanLvl  PortDimensions=1
  TelmClimaExtVentnReqFromExtSrc_TelmClimaExtVentnReqFromExtSrc : Outport  Port=61  OutDataTypeStr=Enum: OnOffNoReq  PortDimensions=1
  TelmClimaExtVentnTempRangeFromExtSrc_TelmClimaExtVentnTempRangeFromExtSrc : Outport  Port=62  OutMin=-256  OutMax=255.9  OutDataTypeStr=T2  PortDimensions=1
  TelmClimaExtVentnTSpSpclFromExtSrc_TelmClimaExtVentnTSpSpclFromExtSrc : Outport  Port=63  OutDataTypeStr=Enum: HmiCmptmtTSpSpcl  PortDimensions=1
  TelmClimaTmrFromExtSrc_TelmClimaTmrFromExtSrc : Outport  Port=64  OutDataTypeStr=Ti33  PortDimensions=1
  TelmSeatDrvrHeatReqFromExsrc_TelmSeatDrvrHeatReqFromExsrc : Outport  Port=65  OutDataTypeStr=Enum: TelmSeatClimaLvlExsrc  PortDimensions=1
  TelmSeatDrvVentnReqFromExsrc_TelmSeatDrvVentnReqFromExsrc : Outport  Port=66  OutDataTypeStr=Enum: TelmSeatClimaLvlExsrc  PortDimensions=1
  TelmSeatPassHeatReqFromExsrc_TelmSeatPassHeatReqFromExsrc : Outport  Port=67  OutDataTypeStr=Enum: TelmSeatClimaLvlExsrc  PortDimensions=1
  TelmSeatPassVentnReqFromExsrc_TelmSeatPassVentnReqFromExsrc : Outport  Port=68  OutDataTypeStr=Enum: TelmSeatClimaLvlExsrc  PortDimensions=1
  TelmSeatSecLeHeatReqFromExsrc_TelmSeatSecLeHeatReqFromExsrc : Outport  Port=69  OutDataTypeStr=Enum: TelmSeatClimaLvlExsrc  PortDimensions=1
  TelmSeatSecLeVentnReqFromExsrc_TelmSeatSecLeVentnReqFromExsrc : Outport  Port=70  OutDataTypeStr=Enum: TelmSeatClimaLvlExsrc  PortDimensions=1
  TelmSeatSecRiHeatReqFromExsrc_TelmSeatSecRiHeatReqFromExsrc : Outport  Port=71  OutDataTypeStr=Enum: TelmSeatClimaLvlExsrc  PortDimensions=1
  TelmSeatSecRiVentnReqFromExsrc_TelmSeatSecRiVentnReqFromExsrc : Outport  Port=72  OutDataTypeStr=Enum: TelmSeatClimaLvlExsrc  PortDimensions=1
  TmrForPrkgClima_TmrForPrkgClima : Outport  Port=73  OutDataTypeStr=CarTiGlb  PortDimensions=1
  WarnForEgyAllwdLimdDly_WarnForEgyAllwdLimdDly : Outport  Port=74  OutDataTypeStr=Boolean  PortDimensions=1
  TelmBookSeatHeatClimalReqDrvHeatClimaLvlFromExtSrc_TelmBookSeatHeatClimalReqDrvHeatClimaLvlFromExtSrc : Outport  Port=75  OutDataTypeStr=Enum: TelmSeatClimaLvlExsrc  PortDimensions=1
  TelmBookSeatHeatClimalReqPassHeatClimaLvlFromExtSrc_TelmBookSeatHeatClimalReqPassHeatClimaLvlFromExtSrc : Outport  Port=76  OutDataTypeStr=Enum: TelmSeatClimaLvlExsrc  PortDimensions=1
  TelmBookSeatHeatClimalReqSecLeHeatClimaLvlFromExtSrc_TelmBookSeatHeatClimalReqSecLeHeatClimaLvlFromExtSrc : Outport  Port=77  OutDataTypeStr=Enum: TelmSeatClimaLvlExsrc  PortDimensions=1
  TelmBookSeatHeatClimalReqSecRiHeatClimaLvlFromExtSrc_TelmBookSeatHeatClimalReqSecRiHeatClimaLvlFromExtSrc : Outport  Port=78  OutDataTypeStr=Enum: TelmSeatClimaLvlExsrc  PortDimensions=1
  TelmBookSeatHeatClimalReqThrdLeHeatClimaLvlFromExtSrc_TelmBookSeatHeatClimalReqThrdLeHeatClimaLvlFromExtSrc : Outport  Port=79  OutDataTypeStr=Enum: TelmSeatClimaLvlExsrc  PortDimensions=1
  TelmBookSeatHeatClimalReqThrdRiHeatClimaLvlFromExtSrc_TelmBookSeatHeatClimalReqThrdRiHeatClimaLvlFromExtSrc : Outport  Port=80  OutDataTypeStr=Enum: TelmSeatClimaLvlExsrc  PortDimensions=1
  TelmBookSeatVentnClimalReqDrvVentnClimaLvlFromExtSrc_TelmBookSeatVentnClimalReqDrvVentnClimaLvlFromExtSrc : Outport  Port=81  OutDataTypeStr=Enum: TelmSeatClimaLvlExsrc  PortDimensions=1
  TelmBookSeatVentnClimalReqPassVentnClimaLvlFromExtSrc_TelmBookSeatVentnClimalReqPassVentnClimaLvlFromExtSrc : Outport  Port=82  OutDataTypeStr=Enum: TelmSeatClimaLvlExsrc  PortDimensions=1
  TelmBookSeatVentnClimalReqSecLeVentnClimaLvlFromExtSrc_TelmBookSeatVentnClimalReqSecLeVentnClimaLvlFromExtSrc : Outport  Port=83  OutDataTypeStr=Enum: TelmSeatClimaLvlExsrc  PortDimensions=1
  TelmBookSeatVentnClimalReqSecRiVentnClimaLvlFromExtSrc_TelmBookSeatVentnClimalReqSecRiVentnClimaLvlFromExtSrc : Outport  Port=84  OutDataTypeStr=Enum: TelmSeatClimaLvlExsrc  PortDimensions=1
  TelmBookSeatVentnClimalReqThrdLeVentnClimaLvlFromExtSrc_TelmBookSeatVentnClimalReqThrdLeVentnClimaLvlFromExtSrc : Outport  Port=85  OutDataTypeStr=Enum: TelmSeatClimaLvlExsrc  PortDimensions=1
  TelmBookSeatVentnClimalReqThrdRiVentnClimaLvlFromExtSrc_TelmBookSeatVentnClimalReqThrdRiVentnClimaLvlFromExtSrc : Outport  Port=86  OutDataTypeStr=Enum: TelmSeatClimaLvlExsrc  PortDimensions=1
  TelmDefrostReqFromExsrc_TelmDefrostReqFromExsrc : Outport  Port=87  OutDataTypeStr=Enum: OnOffNoReq  PortDimensions=1
  TempRangeFromExtSrc_TempRangeFromExtSrc : Outport  Port=88  OutMin=-256  OutMax=255.9  OutDataTypeStr=T2  PortDimensions=1
  wires:
  AmbTFildForClimaNgcc_AmbTFildForClima/1 -> PrkgClimaPreProcMgr_Runnable_sys/1(AmbTFildForClimaNgcc_AmbTFildForClima_read)
  AmbTFildForClimaQf_AmbTFildForClimaQf/1 -> PrkgClimaPreProcMgr_Runnable_sys/2(AmbTFildForClimaQf_AmbTFildForClimaQf_read)
  CarTiIntForClima_CarTiIntForClima/1 -> PrkgClimaPreProcMgr_Runnable_sys/3(CarTiIntForClima_CarTiIntForClima_read)
  ClimaCfgForClimaCtrlMan1_ClimaCfgForClimaCtrlMan1/1 -> PrkgClimaPreProcMgr_Runnable_sys/4(ClimaCfgForClimaCtrlMan1_ClimaCfgForClimaCtrlMan1_read)
  ClimaCfgForComIfGenn2ForTelm1_ClimaCfgForComIfGenn2ForTelm1/1 -> PrkgClimaPreProcMgr_Runnable_sys/5(ClimaCfgForComIfGenn2ForTelm1_ClimaCfgForComIfGenn2ForTelm1_read)
  ClimaCfgForHeatrTyp1_ClimaCfgForHeatrTyp1/1 -> PrkgClimaPreProcMgr_Runnable_sys/6(ClimaCfgForHeatrTyp1_ClimaCfgForHeatrTyp1_read)
  ClimaCfgForHvacHeatrElecFrntPrsnt1_ClimaCfgForHvacHeatrElecFrntPrsnt1/1 -> PrkgClimaPreProcMgr_Runnable_sys/7(ClimaCfgForHvacHeatrElecFrntPrsnt1_ClimaCfgForHvacHeatrElecFrntPrsnt1_read)
  ClimaCfgForSeatHeatgForRowFirstPrsnt1_ClimaCfgForSeatHeatgForRowFirstPrsnt1/1 -> PrkgClimaPreProcMgr_Runnable_sys/8(ClimaCfgForSeatHeatgForRowFirstPrsnt1_ClimaCfgForSeatHeatgForRowFirstPrsnt1_read)
  ClimaCfgForSteerWhlHeatgPrsnt1_ClimaCfgForSteerWhlHeatgPrsnt1/1 -> PrkgClimaPreProcMgr_Runnable_sys/9(ClimaCfgForSteerWhlHeatgPrsnt1_ClimaCfgForSteerWhlHeatgPrsnt1_read)
  ClimaCfgForVehElec1_ClimaCfgForVehElec1/1 -> PrkgClimaPreProcMgr_Runnable_sys/10(ClimaCfgForVehElec1_ClimaCfgForVehElec1_read)
  ClimaCfgForVehHyb1_ClimaCfgForVehHyb1/1 -> PrkgClimaPreProcMgr_Runnable_sys/11(ClimaCfgForVehHyb1_ClimaCfgForVehHyb1_read)
  ClimaCfgForVrntOfVehSysElec1_ClimaCfgForVrntOfVehSysElec1/1 -> PrkgClimaPreProcMgr_Runnable_sys/12(ClimaCfgForVrntOfVehSysElec1_ClimaCfgForVrntOfVehSysElec1_read)
  ClimaRqrd1ConnGen2_ClimaRqrd1ConnGen2/1 -> PrkgClimaPreProcMgr_Runnable_sys/13(ClimaRqrd1ConnGen2_ClimaRqrd1ConnGen2_read)
  ClimaRqrdFromRem1_ClimaRqrdFromRem1/1 -> PrkgClimaPreProcMgr_Runnable_sys/14(ClimaRqrdFromRem1_ClimaRqrdFromRem1_read)
  ClimaStrtTi_ClimaStrtTi/1 -> PrkgClimaPreProcMgr_Runnable_sys/15(ClimaStrtTi_ClimaStrtTi_read)
  ClimaWarn_ClimaWarn/1 -> PrkgClimaPreProcMgr_Runnable_sys/16(ClimaWarn_ClimaWarn_read)